simulink model "MCVT_Integration"  (R2020a)
settings: Solver=ode8  StartTime=0.0  StopTime=T_end  FixedStep=dt  RelTol=1e-3  EnableMultiTasking=on
values from neighbouring files (symbols this model uses):
  assumed_power_voltage = 1.25e2    (= 125)    [MCVT_Input_File.m]    (also in MCVT_Run_QuickCheck.m)
  battery_power_toggle = 1    [MCVT_Input_File.m]    (also in MCVT_Run_QuickCheck.m)
  battery_trickle_charge_param = 0.1    [MCVT_Input_File.m]    (also in MCVT_Run_QuickCheck.m)
  battery_trickle_leakage_param = 0.01    [MCVT_Input_File.m]    (also in MCVT_Run_QuickCheck.m)
  damage_case_id = 3    [MCVT_Input_File.m]    (also in MCVT_Run_QuickCheck.m = 1)
  dt = 1/fs    (= 0.001)    [MCVT_Run_QuickCheck.m]    (also in MCVT_Sim_Set.m)
  dt_2 = 1/fs_2    (= 0.001)    [MCVT_Run_QuickCheck.m]    (also in MCVT_Sim_Set.m)
  dt_3 = 1/fs_3    (= 0.001)    [MCVT_Run_QuickCheck.m]    (also in MCVT_Sim_Set.m)
  dt_5 = 1/fs_5    (= 0.005)    [MCVT_Run_QuickCheck.m]    (also in MCVT_Sim_Set.m)
  dt_8 = 1/fs_8    (= 0.05)    [MCVT_Run_QuickCheck.m]    (also in MCVT_Sim_Set.m)
  dt_9 = 1/fs_9    (= 0.001)    [MCVT_Run_QuickCheck.m]    (also in MCVT_Sim_Set.m)
  dust_met_duration = 3    [MCVT_Input_File.m]    (also in MCVT_Run_QuickCheck.m = 2)
  ECLSS_indoor_set_temperature = 298.15    [MCVT_Input_File.m]    (also in MCVT_Run_QuickCheck.m)
  energy_store_max_o = 150    [MCVT_Input_File.m]    (also in MCVT_Run_QuickCheck.m)
  energy_store_o = 120    [MCVT_Input_File.m]    (also in MCVT_Run_QuickCheck.m)
  fs = 1000    [MCVT_Run_QuickCheck.m]    (also in MCVT_Sim_Set.m)
  gain_charge_param = 1    [MCVT_Input_File.m]    (also in MCVT_Run_QuickCheck.m)
  ground_temperature = 373    [MCVT_Habitat_Design_Input_File.m]    (also in MCVT_Run_QuickCheck.m)
  I_max_charge = 800    [MCVT_Input_File.m]    (also in MCVT_Run_QuickCheck.m)
  I_max_discharge = 800    [MCVT_Input_File.m]    (also in MCVT_Run_QuickCheck.m)
  initial_pressure = 1e5    (= 100000)    [MCVT_Input_File.m]    (also in MCVT_Run_QuickCheck.m)
  initial_temperature = 295    [MCVT_Input_File.m]    (also in MCVT_Run_QuickCheck.m)
  interior_leak_rate = 0.00415    [MCVT_Input_File.m]    (also in MCVT_Run_QuickCheck.m)
  launch_dust_accum_start_time = 10    [MCVT_Input_File.m]    (also in MCVT_Run_QuickCheck.m = 2)
  launch_dust_accum_stop_time = 12    [MCVT_Input_File.m]    (also in MCVT_Run_QuickCheck.m = 3)
  meteorite_impact_time = 2    [MCVT_Input_File.m]    (also in MCVT_Run_QuickCheck.m = 1)
  nuclear_power_toggle = 1    [MCVT_Input_File.m]    (also in MCVT_Run_QuickCheck.m)
  print_interval = 30    [MCVT_Input_File.m]
  SOC_lower_thres = 0.5    [MCVT_Input_File.m]    (also in MCVT_Run_QuickCheck.m)
  SOC_upper_thres = 0.9    [MCVT_Input_File.m]    (also in MCVT_Run_QuickCheck.m)
  solar_power_toggle = 1    [MCVT_Input_File.m]    (also in MCVT_Run_QuickCheck.m)
  space_temperature = 4    [MCVT_Habitat_Design_Input_File.m]    (also in MCVT_Run_QuickCheck.m)
  Start_angle = 90    [MCVT_Input_File.m]    (also in MCVT_Run_QuickCheck.m)
undefined symbols (not defined in the file or in its folder): A_flow_restrictor, ACTIVITY_LOOKUP_TABLE, air, Aref, Bmap, Bmap2, Bmap3, comms, DiscIntNeverNeededParam, DmgSPL, dust_parameters, ECLSS_dust_damage_repair_time, ECLSS_heater_capacity, ECLSS_IPS_restrictor, ECLSS_IPS_setpointPressure, ECLSS_paint_damage_repair_time, ECLSS_radiatorLoop_discreteNumber, ECLSS_Vst, emptyActivity, expected_heat_add, FI, habitat, HIEM2SMM, HIEM2STM, HIEMDamageElements, impact_numbering, initial_SPLT_damage_index, input_air, input_Cond, input_CP, input_discretization, input_EV, input_Evap, input_radiatorLoop, K_ECLSS_dust_single, K_ECLSS_paint_single, K_Structure_damage_single, Kref, kW_to_W, meteoroidDustDep, mFRF, ndelays, nominalDustDep, noverlap, nuclear_toggle_gain, output_limits, panel_paint0, Ps0, reference_temperature, RepairTime_ECLSS_factor, Sensor_Location_SMM, SMM_T0, SMM_T1, SMM_T2, SMM_T3, SMM_T4, SMM_T4_0, SMM_T4_1, SMM_T4_2, solar_FDD_K ... (+73)
libraries: neural x350, simulink x39, xpcutilitieslib x7, xpcrtudplib x7, dspstat3 x1
(part 41 of 43)

== matlab function MCVT_Integration/Sys8 - Interior Environment/Habitat Interior Environment Model/Temperature Sensors/ Resistance Temperature Detector2/R vs T ==
  data: R input; T output
function T = fcn(R)

T = 1.76520388802152e-09 * R^4 - 2.98990866289637e-07 * R^3 + 0.000987249006463873 * R^2 ...
    + 2.36178487086887 * R - 245.924688605077;

end

== matlab function MCVT_Integration/Sys8 - Interior Environment/Habitat Interior Environment Model/Temperature Sensors/ Resistance Temperature Detector2/RTD with Self-heat Error/MATLAB Function ==
  data: I input; R input; U output
function U = fcn(I, R)

U = I * R;     % Operating current = 1mA
               % Voltage output U = I*R

== matlab function MCVT_Integration/Sys8 - Interior Environment/Habitat Interior Environment Model/Temperature Sensors/ Resistance Temperature Detector2/RTD without Self-heat Error/MATLAB Function ==
  data: I input; I output; R input; U output
function [I,U]= fcn(I,R)

U = I * R;     % Operating current = 1mA
               % Voltage output U = I*R

== matlab function MCVT_Integration/Sys8 - Interior Environment/Habitat Interior Environment Model/Temperature Sensors/ Resistance Temperature Detector2/Self-heat Calculation/  T vs R  ==
  data: R output; T input
function R = fcn(T)

if T >= 0
    R = 100*(1+3.9083*10^(-3)*T-5.775*10^(-7)*T^2);
else
    R = 100*(1+3.9083*10^(-3)*T-5.775*10^(-7)*T^2-4.183*10^(-12)*(T-100)*T^3);
end

== matlab function MCVT_Integration/Sys8 - Interior Environment/Habitat Interior Environment Model/Temperature Sensors/ Resistance Temperature Detector2/Switch ON//OFF Control Block/Switch OFF/MATLAB Function1 ==
  data: u input; y output; Time input
function y = fcn(u,Time)

if Time>=0
    u = NaN;
end

y = u;

== matlab function MCVT_Integration/Sys8 - Interior Environment/Habitat Interior Environment Model/Temperature Sensors/ Resistance Temperature Detector2/T vs R ==
  data: R output; T input
function R = fcn(T)

if T >= 0
    R = 100*(1+3.9083*10^(-3)*T-5.775*10^(-7)*T^2);
else
    R = 100*(1+3.9083*10^(-3)*T-5.775*10^(-7)*T^2-4.183*10^(-12)*(T-100)*T^3);
end

== matlab function MCVT_Integration/Sys8 - Interior Environment/Habitat Interior Environment Model/Temperature Sensors/ Switch ON//OFF Control Number ==
  data: Power input; Switch output
function Switch = Switch1(Power)

if Power == 0
    Switch = 2;
else
    Switch = 1;
end

end

== matlab function MCVT_Integration/Sys8 - Interior Environment/Habitat Interior Environment Model/Temperature Sensors/ Switch ON//OFF Control Number1 ==
  data: Power input; Switch output
function Switch = Switch2(Power)

if Power == 0
    Switch = 2;
else
    Switch = 1;
end

end

== matlab function MCVT_Integration/Sys8 - Interior Environment/Habitat Interior Environment Model/Temperature Sensors/ Switch ON//OFF Control Number2 ==
  data: Power input; Switch output
function Switch = Switch3(Power)

if Power == 0
    Switch = 2;
else
    Switch = 1;
end

end

== matlab function MCVT_Integration/Sys3 - Power System/Power Generation Subsystem1/Nuclear Power Generation/SP-100 Power Generation/	Power Generation/Sensor Models/Cold Junction Temperature Sensing Model4/ Resistance Temperature Detector/R vs T ==
  data: R input; T output
function T = fcn(R)

T = 1.76520388802152e-09 * R^4 - 2.98990866289637e-07 * R^3 + 0.000987249006463873 * R^2 ...
    + 2.36178487086887 * R - 245.924688605077;

end

== matlab function MCVT_Integration/Sys2 - Structural System/Sys2_Structural_System/Sim FDD Damage Detection/MATLAB Function ==
  data: u input; xtrue output
function xtrue = TobinaryHS_damage(u)
    if u > 0.5
        xtrue = 1;
    else
        xtrue = 0;
    end
end

== matlab function MCVT_Integration/Sys3 - Power System/Power Generation Subsystem1/Nuclear Power Generation/SP-100 Power Generation/	Power Generation/Sensor Models/Cold Junction Temperature Sensing Model4/ Resistance Temperature Detector/RTD with Self-heat Error/MATLAB Function ==
  data: I input; R input; U output
function U = fcn(I, R)

U = I * R;     % Operating current = 1mA
               % Voltage output U = I*R

== matlab function MCVT_Integration/Sys8 - Interior Environment/Habitat Interior Environment Model/Temperature Sensors/Sensor Input to CDCM ==
  data: ECLSS input; Air_Temp_Sensed output; Zenith input; LeftBottom input
function Air_Temp_Sensed = Sensor_Placement(ECLSS,Zenith,LeftBottom)

a = ECLSS(1,1);
b = Zenith(1,1);
c = LeftBottom(1,1);

Air_Temp_Sensed = ones(3,1);

Air_Temp_Sensed(1,1) = a;
Air_Temp_Sensed(2,1) = b;
Air_Temp_Sensed(3,1) = c;

end

== matlab function MCVT_Integration/Sys8 - Interior Environment/Habitat Interior Environment Model/Temperature Sensors/Sensor Placement ==
  data: Air_Temp input; ECLSS output; Zenith output; LeftBottom output
function [ECLSS,Zenith,LeftBottom] = Sensor_Placement(Air_Temp)

Locations = [65 10 19];

Temp_to_Sensor = Air_Temp(Locations,1);

ECLSS = Temp_to_Sensor(1,1);
Zenith = Temp_to_Sensor(2,1);
LeftBottom = Temp_to_Sensor(3,1);

end

== matlab function MCVT_Integration/Sys3 - Power System/Power Generation Subsystem1/Nuclear Power Generation/SP-100 Power Generation/	Power Generation/Sensor Models/Cold Junction Temperature Sensing Model4/ Resistance Temperature Detector/RTD without Self-heat Error/MATLAB Function ==
  data: I input; I output; R input; U output
function [I,U]= fcn(I,R)

U = I * R;     % Operating current = 1mA
               % Voltage output U = I*R

== matlab function MCVT_Integration/Sys3 - Power System/Power Generation Subsystem1/Nuclear Power Generation/SP-100 Power Generation/	Power Generation/Sensor Models/Cold Junction Temperature Sensing Model4/ Resistance Temperature Detector/Self-heat Calculation/  T vs R  ==
  data: R output; T input
function R = fcn(T)

if T >= 0
    R = 100*(1+3.9083*10^(-3)*T-5.775*10^(-7)*T^2);
else
    R = 100*(1+3.9083*10^(-3)*T-5.775*10^(-7)*T^2-4.183*10^(-12)*(T-100)*T^3);
end

== matlab function MCVT_Integration/Sys3 - Power System/Power Generation Subsystem1/Nuclear Power Generation/SP-100 Power Generation/	Power Generation/Sensor Models/Cold Junction Temperature Sensing Model4/ Resistance Temperature Detector/Switch ON//OFF Control Block/Switch OFF/MATLAB Function1 ==
  data: u input; y output; Time input
function y = fcn(u,Time)

if Time>=0
    u = NaN;
end

y = u;

== matlab function MCVT_Integration/Sys3 - Power System/Power Generation Subsystem1/Nuclear Power Generation/SP-100 Power Generation/	Power Generation/Sensor Models/Cold Junction Temperature Sensing Model4/ Resistance Temperature Detector/T(Celcuis) vs R ==
  data: R output; T input
function R = fcn(T)

if T >= 0
    R = 100*(1+3.9083*10^(-3)*T-5.775*10^(-7)*T^2);
else
    R = 100*(1+3.9083*10^(-3)*T-5.775*10^(-7)*T^2-4.183*10^(-12)*(T-100)*T^3);
end

== matlab function MCVT_Integration/Sys3 - Power System/Power Generation Subsystem1/Nuclear Power Generation/SP-100 Power Generation/	Power Generation/Sensor Models/block/MATLAB Function2 ==
  data: y_Tf output; y_process_scale_lower input; y_Tb1 output; y_Tb2 output; y_Tc2 output
function [y_Tf, y_Tb1, y_Tb2, y_Tc2] = fcn(y_process_scale_lower)
    y_Tf = y_process_scale_lower(:,1);
    y_Tb1 = y_process_scale_lower(:,2);
    y_Tb2 = y_process_scale_lower(:,3);
    y_Tc2 = y_process_scale_lower(:,4);
end

== matlab function MCVT_Integration/Sys3 - Power System/Power Generation Subsystem1/Solar Power Generation/MATLAB Function1 ==
  data: u input; y output
function y = ToTrueHealth(u)
    if 0 <= u && u < 0.05
        y = 1; % healthy
    elseif 0.05 <= u && u < 0.3
        y = 2; % minor
    elseif 0.3 <= u && u < 0.7
        y = 3; % medium
    else %0.7 <= u && u <= 1
        y = 4; % major
    end

== matlab function MCVT_Integration/Sys3 - Power System/Power Generation Subsystem1/Nuclear Power Generation/SP-100 Power Generation/FDD Iterative Online Residual Analysis1/ Digital Twin of SP100/Residual Generation/Compare to Nominal/CUSUM5/cusum ==
  data: intervention input; cusum_stat1 output; time input; time_step input; cusum_stat1 input; intervention_previous output; intervention_previous input
function [cusum_stat1,intervention_previous]  = fcn(intervention, time, time_step, cusum_stat1,intervention_previous)

[cusum_stat1,intervention_previous]  = cusum(intervention, time, time_step, cusum_stat1,intervention_previous);

end

== matlab function MCVT_Integration/Sys3 - Power System/Power Generation Subsystem1/Nuclear Power Generation/SP-100 Power Generation/FDD Iterative Online Residual Analysis1/ Digital Twin of SP100/Residual Generation/FDD_toggle ==
  data: FDD_signal input; FDD_signal_sent output; nuclear_power_toggle input; battery_power_toggle input
function FDD_signal_sent = FDD_toggle(FDD_signal, nuclear_power_toggle,...
    battery_power_toggle)

FDD_signal_sent = FDD_toggle(FDD_signal, nuclear_power_toggle,...
    battery_power_toggle);

end

== matlab function MCVT_Integration/Sys3 - Power System/Power Generation Subsystem1/Power Distribution and Storage System/Subsystem/power_gen_allocation ==
  data: G_solar_temporary input; G_solar_power output; P_out_solar input; V input; I_tot input; energy_stored input; max_storage input; time_step input; G_nuclear input; I_nuclear input; SOC_lower_thresh input; energy_stored output; time_to_discharge output; SOC_upper_thresh input; SOC output; G_nuclear_power output; battery_use_status output; leakage_parameter input; trickle_charge_parameter input; charge_parameter input; I_max_charge input; I_max_discharge input; total_power_avail output; load_shed_status output; I_battery output; sub2_request input; sub5_request input; sub8_request input; battery_power_toggle input
function [G_solar_power,energy_stored,time_to_discharge,SOC,...
    G_nuclear_power,battery_use_status,total_power_avail,...
    load_shed_status,I_battery]=power_gen_allocation(G_solar_temporary,...
    P_out_solar,V,I_tot,energy_stored,max_storage,time_step, G_nuclear,...
    I_nuclear,SOC_lower_thresh,SOC_upper_thresh, leakage_parameter,...
    trickle_charge_parameter,charge_parameter,I_max_charge,...
    I_max_discharge,sub2_request,sub5_request,sub8_request,...
    battery_power_toggle)


[G_solar_power,energy_stored,time_to_discharge,SOC,...
    G_nuclear_power,battery_use_status,total_power_avail,...
    load_shed_status,I_battery]=power_gen_allocation(G_solar_temporary,...
    P_out_solar,V,I_tot,energy_stored,max_storage,time_step, G_nuclear,...
    I_nuclear,SOC_lower_thresh,SOC_upper_thresh, leakage_parameter,...
    trickle_charge_parameter,charge_parameter,I_max_charge,...
    I_max_discharge,sub2_request,sub5_request,sub8_request,...
    battery_power_toggle);


end

== matlab function MCVT_Integration/Sys3 - Power System/Power Generation Subsystem1/Power Distribution and Storage System/Subsystem/MATLAB Function1 ==
  data: I_nuc input; G_nuc output; I_tot input
function G_nuc  = fcn(I_nuc,I_tot)
if I_nuc ~= 0
    G_nuc = min(1, I_tot/I_nuc);
else
    G_nuc = 0;
end
end

== matlab function MCVT_Integration/Sys3 - Power System/Power Generation Subsystem1/Power Distribution and Storage System/Subsystem2/Dummy Electrical Power Consuming Equipment3/MATLAB Function ==
  data: p_avail input; p_desired output
function p_desired  = fcn(p_avail)

  % A system that has two modes and enters mode 2 if p_avail < 20
  if (p_avail < 20)
        p_desired = p_avail;
  else
        p_desired = 50;
  end

end

== matlab function MCVT_Integration/Sys2 - Structural System/Sys2_Structural_System/Structural Thermal Model 2.2/STM Temperature Sensors/ Resistance Temperature Detector/Power Consumption ==
  data: Supply input; kWUsage1 output; Switch input
function kWUsage1 = Power_Consumption(Supply,Switch)
if Switch >= 1
    Voltage = 5.5; %Volts
    Current = 0.000001; %10 microAmperes
    kWUsage1 = ones(1,1);
    
    kWUsage1 = (Voltage * Current)/1000;
    
    if kWUsage1 >= Supply
        kWUsage1 = Supply;
    end
else
    kWUsage1 = 0;
end
end

== matlab function MCVT_Integration/Sys3 - Power System/Power Generation Subsystem1/printRepair ==
  data: x input
function  printRepair(x)


if x(1)>0
    disp('        Solar Panel is Cleaned');
end   


end

== matlab function MCVT_Integration/Sys3 - Power System/Power Generation Subsystem1/printRepair1 ==
  data: x input
function printRepair(x)


if x(1)>0
    disp('        Nuclear Radiator is Cleaned');
end   


end

== matlab function MCVT_Integration/Sys2 - Structural System/Sys2_Structural_System/Fault Detection and Diagnosis (FDD) 2.3/Accelerometer/Accelerometer/ Switch ON//OFF Control Number ==
  data: p input; y output
function y = fcn(p)

if p >= 1.2*10^(-4)*3
    u = 1;
else
    u = 2;
end

y = u;

== matlab function MCVT_Integration/Sys8 - Interior Environment/power1 ==
  data: Y output; x input
function Y = poweredit(x)

Y=max(5.5e-9*3, x);

end

== matlab function MCVT_Integration/Sys2 - Structural System/Sys2_Structural_System/Structural Mechanical 2.1/MATLAB Function ==
  data: u input; y output
function y = fcn(u)

y = norm(u);

end

== matlab function MCVT_Integration/Sys2 - Structural System/power2 ==
  data: Y output; x input
function Y = poweredit(x)

Y=max(0.0036, x);

end

== matlab function MCVT_Integration/Sys9 - Exterior Environment/Exterior Environment/Reads cartesian coordinates ==
  data: pos_out output
function pos_out = Coordinate_reader()
    
CARCOOM=coder.load ('SPLxyz.mat'); %Loads the user provided cartesian coordinates into the program%
pos_out=CARCOOM.STMregxyz;

end

== matlab function MCVT_Integration/Sys5 - ECLSS/Human//System 5/Human Heat Model V2 ==
  data: dt input; activity_id input; heat_profile_final output; Location_id input; r output; theta output
function [heat_profile_final, r, theta]  =...
    eclss_human_model(dt,activity_id,Location_id)


%Activity 1: SleepHeat = 317 kJ/hr
%Activity 2: NominalHeat = 500 kJ/hr
%Activity 3: Exercise1 = 1206 kJ/hr
%Activity 4: Exercise2 = 2974 kJ/hr
%Activity 5: Exercise3 = 2005 kJ/hr
%Activity 6: Exercise4 = 1078 kJ/hr
%Activity 7: Exercise5 = 865 kJ/hr
%Activity 8: Exercise6 = 751 kJ/hr
%Activity 9: Other = 0 kJ/hr


activity_heat = [317 500 1206 2974 2005 1078 865 751 0]; %kJ/hr
heat_per_hr = activity_heat(floor(activity_id)); %kJ/hr
heat_profile = heat_per_hr/(60*60)*dt*1000; %J/dt
heat_profile_final = heat_profile * Location_id(1);
r = Location_id(2); % feet
theta = Location_id(3); % degrees

== matlab function MCVT_Integration/Sys 6 - Health Management and Agents/Decision Maker/MATLAB Function ==
  data: suggested_activity_id input; pgm output; lookup_table input; time input
function pgm = acvt_lookup_function(suggested_activity_id, lookup_table, time)
%         #=================================================#
%         #    Code developed for the RETH-Institute        #
%         #    By: Murali Krishnan Rajasekharan Pillai      #
%         #    PhD Student @ Purdue University              #
%         #    Funded by: NASA                              #
%         #    Last Modified: July 29, 2021                 #
%         #=================================================#
% 
%         Purpose: Function that looks-up activity definitions from a look-up table
%         specified in the `InitAgentModel.m`

    valid_activity_ids = [1, 2, 3, 4, 5, -1];
    valid_active_acvt_ids = [1, 2, 3, 4, 5];
    assert(ismember(suggested_activity_id, valid_activity_ids), "Invalid Activity ID");
    if ismember(suggested_activity_id, valid_active_acvt_ids)
        pgm = squeeze(lookup_table(suggested_activity_id, :, :));
    else
        pgm = squeeze(lookup_table(6, :, :));
    end
    
    if time == 35 && suggested_activity_id == -9999
        pgm = squeeze(lookup_table(4, :, :));
    end
    if time == 30 && suggested_activity_id == -9999
        pgm = squeeze(lookup_table(5, :, :));
    end
end

== matlab function MCVT_Integration/Sys2 - Structural System/Sys2_Structural_System/Fault Detection and Diagnosis (FDD) with Binary Classifer/Post-processing Block ==
  data: u input; y output; t input; repair_begin input; impact_begin input
function y = fcn(u,t,repair_begin,impact_begin)

persistent count repair_begin_time

if isempty(count) && isempty(repair_begin_time)
    count = 0;
    repair_begin_time = 10000;
end

if ~isnan(repair_begin) && count == 0
    repair_begin_time = repair_begin;
    count = 1;
end

if t >= 0 && t < 7 + 0.002
    u(:,:) = nan;
else
    if t >= impact_begin + 0.002 && t < impact_begin + 7 + 0.002
        u(:,:) = nan;
    else
        if t >= ceil(repair_begin_time) + 0.002 && t < ceil(repair_begin_time) + 7 + 0.002
            % for impact location [-2.4807    1.0619    1.0622]
            % repair happen 39.4140s, reapir complete 40.4130
            % for other impact locations
            % repair happen 33.4120s, reapir complete 34.4110
            u(1:8,:) = nan;
        end
    end
end

% For segment 5
if t >= ceil(repair_begin_time) + 0.002 && t < ceil(repair_begin_time) + 13 + 0.002
    u(9:10,:) = nan;
end
            
y = u;

== matlab function MCVT_Integration/Sys2 - Structural System/Sys2_Structural_System/Fault Detection and Diagnosis (FDD) with Binary Classifer/Find repair start time ==
  data: u input; y output; t input
function y = fcn(u,t)

persistent count

if isempty(count)
    count = 0;
end

if u == 1 && count == 0
    y = t;
    count = 1;
else
    y = nan;
    
end

== matlab function MCVT_Integration/Sys5 - ECLSS/Active Thermal Control System (ATCS)/Faulty Panels/MATLAB Function3 ==
  data: impact_numbering input; N input; impact input; L_dust output size=[1,50]
function L_dust  = Damage_factory_Dust(impact_numbering, N, impact)
%% Documentation
% Purpose: Assign which panels to be damaged when there is a meteorite
% impact
% Date Created: 20 June 2020
% Date Last Modified: 27 July 2021
% Modeler Name: Jaewon Park (Purdue)
% Funding Acknowledgement: Funded by the NASA RETHi Project (80NSSC19K1076)

% Version Number: MCVT v1.4

% Subsystem Connections: receives input from subsystem 8.

% INPUTS %
%   - impact_numbering: impact scenario lower and upper bounds, type: integer, [1x2]
%   - impact: impact severity, type: integer
%   - N: total number of panel subsections, type: integer
    
% OUTPUTS %
%   - L_dust: list of subsection indices which should be damaged, [1x50], type: integer 

% Function Dependencies: No dependency

% No Data Depenencies
%% assign damaged panels by meteorite impact 
min_impact = impact_numbering(1);                                                                     % minimum impact severity         
max_impact = impact_numbering(2);                                                                     % maximum impact severity
n_panels_impact = 20;                                                               % maximum number of panels that can be impacted (To do) update needed as we move on from 60s simulation limit
mapping_ratio_impact = (impact - min_impact)/max_impact;                            % mapping 
n_to_be_affected_impact = round(n_panels_impact * mapping_ratio_impact);            % number of panels to be affected 
%disp(n_to_be_affected_impact)
temp = sort([randperm(N,n_to_be_affected_impact), zeros(1,N-n_to_be_affected_impact)],'descend');   
L_dust = zeros(1,50);
L_dust = L_dust + temp;                                                             % assign damaged panels
end

== matlab function MCVT_Integration/Sys2 - Structural System/Sys2_Structural_System/Structural Mechanical 2.1/Force Calculation ==
  data: Ext_F output; In_Acc output; fT input; fT_e input; eT input; TIn input; Dom input; PIn input; MQ_Acc input; WN_Acc input; Imp_F input; T_Node input; SMM_T0 parameter; SMM_T1 parameter; fTO output; fT_eO output; eTO output; SMM_T2 parameter; SMM_T4_0 parameter; SMM_T4_1 parameter; SMM_T4_2 parameter
function [Ext_F,In_Acc,fTO,fT_eO,eTO]=Force_Calculation_Sim(fT,fT_e,eT,TIn,Dom,PIn,MQ_Acc,WN_Acc,Imp_F,T_Node,SMM_T0,SMM_T1,SMM_T2,SMM_T4_0,SMM_T4_1,SMM_T4_2)
            

%% This block determine Equivalent force vector from 
%   1. Internal Pressure
%   2. Temperature
%   3. Ground acceleration and White noise
%   4. Impact force

% Inputs from outher models:
%       PIn: Pressure input (Interior env)
%       TIn: Temperature input from STM (profile for Structure)
%       In_Acc: Acceleration Input
%       Imp_F: Impact force input

% Inputs from Main\Workspace
%       SMM_T0,SMM_T1,SMM_T2,SMM_T4_0,SMM_T4_1,SMM_T4_2: Read SysFiles\Subs2\SMM_Readme.txt

% State dependent var for SMM computation:
%       fT: Force vector due to temperature at previous timestep/iteration
%       fT_e: Force matrix (per element) due to temperature at previous timestep/iteration
%       eT: Element number for thermal stress computation at previous timestep/iteration
%       Dom: Domain number

% Outputs:
%       Ext_F: Force vector output to Solver block
%       Ext_A: Acceleration vector output to Solver block
%       fTO: Force vector due to temperature at this timestep/iteration
%       fT_eO: Force matrix (per element) due to temperature at this timestep/iteration
%       eTO: Element no for thermal stress computation at this timestep/iteration

% System D.O.F.
    ndof=3;

  
%% Equivalent nodal force from Internal pressure calculation (High efficiency)

    % Get pressure by averaging internal pressure PIn from Interior environment model
        P=zeros(SMM_T1.nel,1)+mean(PIn);   
            
    % Calculate Force vector due to pressure
        [fS]=Pressure_to_Equivalent_Nodal_Force(SMM_T1.nnp,SMM_T4_1.LM_M(:,:,Dom),SMM_T0.Type,SMM_T0.S_Type,SMM_T2.P_surf,P); % Convert pressure to Nodal force 
          
            
%% Equivalent nodal force from Temperature calculation (Low efficiency) 

    % Summary
        % Thermal stress computation is computationally extensive. 
        % Hence thermal stress for one element "eT" per iteration has been carried out

    % ---------------Construct temperature using mapping vector (One to One)-------------
        % Safe: Can work with any domain
        % STM map temperature for Domain 1. Nodes for Domain 1
            NodesFP=SMM_T4_0.Nodes_M(:,:,1); 
        % Nodes for Domain Dom
            Nodes=SMM_T4_0.Nodes_M(:,:,Dom);
        % Initialize Temperature for Domain Dom    
            T=zeros(SMM_T1.nnp,1)+nanmean(TIn); % Initialize Temperature vector with mean temperature
            
            for n=1:SMM_T1.nnp % Go through all nodes
                for ii=1:SMM_T1.nnp
                    % Distance between Node n of Domain Dom and Node i of Domain NodesFP
                    Diff=norm(Nodes(n,2:4)-NodesFP(ii,2:4));  
                    if abs(Diff)<10^-5  % If difference <Tol
                        if isnan(TIn)==0 % Safety check for input loadings if they become NaN
                            T(n)=TIn(ii);    % Set the Temperature value
                        end
                        break
                    end
                end
            end
                
    % ----------------------------Convert Nodal Temperature to Gauss point-----------------------
        [Tm]=Gauss_Point_Temperature_Matrix_Generator(T,SMM_T0.Type,SMM_T1.Shape,SMM_T4_1.IEN_Colm_M(:,Dom),SMM_T1.nel);   
            
    % -------------------Convert Gauss point temperature to Equivalent nodal force-----------------
        % Subtract the elemental Force vector of element eT from assembled force vector fT
            fT(SMM_T4_1.LM_M(1:SMM_T0.Type(2,eT)*ndof,eT,Dom),1)=fT(SMM_T4_1.LM_M(1:SMM_T0.Type(2,eT)*ndof,eT,Dom),1)-fT_e(1:SMM_T0.Type(2,eT)*ndof,eT);   % Eleminate force due to Temperature for element eT from total force vector fT                    
        % Initialize the elemental force vector for eT     
            fT_e(:,eT)=fT_e(:,eT)*0;     % Initialize FV. Assign 0 at local force vector fT_e for element eT
               
        % Get fT_e, Force vector due to temperature for element eT
            for gp=1:SMM_T0.Type(ndof,eT)
                % fT_e=fT_e+IS_F*delT
                fT_e(1:SMM_T0.Type(2,eT)*ndof,eT)=fT_e(1:SMM_T0.Type(2,eT)*ndof,eT)+SMM_T2.IS_F(1:SMM_T0.Type(2,eT)*ndof,gp,eT)*(Tm(gp,eT)-SMM_T0.Tinit);               
            end
        % Add fT_e to assembled force vector fT
            fT(SMM_T4_1.LM_M(1:SMM_T0.Type(2,eT)*ndof,eT,Dom),1)=fT(SMM_T4_1.LM_M(1:SMM_T0.Type(2,eT)*ndof,eT,Dom),1)+fT_e(1:SMM_T0.Type(2,eT)*ndof,eT);

        % If eT is higher than SMM_T1.nel, reset eT
            eT=eT+1;
            if eT>SMM_T1.nel
               eT=1;
            end

        % Setting output different name then input
            fTO=fT;
            fT_eO=fT_e;
            eTO=eT;

%% Total force vector calculation

    % fT: Thermal
    % SMM_T4_2.fB_M: Gravity
    % fS: Internal pressure
    % L is the D.O.F. left after removing Fixed end condition

    Ext_F=(fT+SMM_T4_2.fB_M(:,Dom)+fS);

%% Equivalent nodal force from Impact force calculation      
    % Convert Impact force perpendicular to node

    % Set the impact force
    if Imp_F>0
        % Normal unit vector of the surface
            Rad=norm([SMM_T4_0.Nodes_M(T_Node,2,Dom) SMM_T4_0.Nodes_M(T_Node,ndof,Dom) SMM_T4_0.Nodes_M(T_Node,4,Dom)]);
        % Set the impact force
            Imp_F=-Imp_F/Rad;
            Ext_F(T_Node*ndof-2,1)=Ext_F(T_Node*ndof-2,1)+Imp_F*SMM_T4_0.Nodes_M(T_Node,2,Dom);
            Ext_F(T_Node*ndof-1,1)=Ext_F(T_Node*ndof-1,1)+Imp_F*SMM_T4_0.Nodes_M(T_Node,ndof,Dom);
            Ext_F(T_Node*ndof,1)=Ext_F(T_Node*ndof,1)+Imp_F*SMM_T4_0.Nodes_M(T_Node,4,Dom);            
    end
            

%% Acceleration computation     

    % Initialize acceleration
        In_Acc=zeros(SMM_T1.neq,1);

    % Set acceleration vector along x direction    
        In_Acc(1:ndof:end-2,1)=ones(SMM_T1.neq/ndof,1)*(MQ_Acc(1)+WN_Acc(1));
    % Set acceleration vector along y direction
        In_Acc(2:ndof:end-1,1)=ones(SMM_T1.neq/ndof,1)*(MQ_Acc(2)+WN_Acc(1));
    % Set acceleration vector along z direction
        In_Acc(3:ndof:end-0,1)=ones(SMM_T1.neq/ndof,1)*(MQ_Acc(3)+WN_Acc(1));            
            
 
end

%% Pressure calculation
function[fS]=Pressure_to_Equivalent_Nodal_Force(nnp,LM,Type,S_Type,P_surf,Ps)

% S_Type contains surface information
    SS=size(S_Type);

% Initialize force vector due to pressure on surface fS        
    fS=zeros(nnp*3,1);

% For all the surfaces
    for ii=1:SS(1)

        SN=S_Type(ii,1);                           % Extract Surface number

        e=S_Type(ii,2);                            % Extract element no

        Pes=Ps(ii,SN);                             % Extract Element Pressure

        fe=P_surf(1:Type(2,e)*3,ii,SN)*Pes;        % Determine Equivalent Nodal Force vector

        fS(LM(1:Type(2,e)*3,e))=fS(LM(1:Type(2,e)*3,e))+fe;     % Put into Total/assembled Force Vector

    end
    

end

%% Gauss point temperature calculation 
function[Tm]=Gauss_Point_Temperature_Matrix_Generator(T,Type,Shape,IEN_Colm,nel)

% Temperature in Expanded format
    Te=T(IEN_Colm);
% Initialize temperature matrix 
    % Rows: Gauss points
    % Column: Element number
    Tm=zeros(max(Type(3,:)),nel);

% Populate Tm for each element    
    count=1;
    for e=1:nel % Element number e

        % Get Temperature at Gauss points for specific element e
            Tm(1:Type(3,e),e)=Shape(1:Type(3,e),1:Type(2,e),Type(1,e))*Te(count:count+Type(2,e)-1,1); 

        % Keep track of Expanded temperature vector
            count=count+Type(2,e);
    end


end

== matlab function MCVT_Integration/Sys3 - Power System/Power Generation Subsystem1/accumulation adjust ==
  data: x input; y output
function y = dust_accumu(x)

y=max(x*500,0);


end

== matlab function MCVT_Integration/Sys3 - Power System/Power Generation Subsystem1/Power Distribution and Storage System/Power Delivered to each EPCE/power_distribution ==
  data: total_power_avail input; power_demands output; power_demands input; status_data output
function [power_demands, status_data]  = power_distribution(...
     total_power_avail,power_demands)

[power_demands, status_data]  = power_distribution(...
     total_power_avail,power_demands);
 
end

== matlab function MCVT_Integration/Sys5 - ECLSS/Active Thermal Control System (ATCS)/Faulty Panels/MATLAB Function4 ==
  data: impact_numbering input; N input; impact input; L_paint output size=[1,50]
function L_paint  = Damage_factory_paint(impact_numbering, N, impact)
%% Documentation
% Purpose: Assign which panels to be damaged when there is a meteorite
% Date Created: 20 June 2020
% Date Last Modified: 27 July 2021
% Modeler Name: Jaewon Park, CJ Pan (Purdue)
% Funding Acknowledgement: Funded by the NASA RETHi Project (80NSSC19K1076)

% Version Number: MCVT v1.4

% Subsystem Connections: receives input from subsystem 8.

% INPUTS %
%   - impact_numbering: impact scenario lower and upper bounds, type: integer, [1x2]
%   - impact: impact severity, type: integer
%   - N: total number of panel subsections, type: integer
    
% OUTPUTS %
%   - L_paint: list of subsection indices which should be damaged, [1x50], type: integer

% Function Dependencies: No dependency

% No Data Depenencies
%% Assign paint damage
min_impact = impact_numbering(1);             % minimum impact severity 
max_impact = impact_numbering(2);             % maximum impact severity 
n_panels_impact = 20;       % maximum number of panels that can be impacted (To do) update needed as we move on from 60s simulation limit
mapping_ratio_impact = (impact - min_impact)/max_impact;    % mapping
n_to_be_affected_impact = round(n_panels_impact * mapping_ratio_impact);    % number of panels to be affected 
%disp(n_to_be_affected_impact)
temp = sort([randperm(N,n_to_be_affected_impact), zeros(1,N-n_to_be_affected_impact)],'descend');
L_paint = zeros(1,50);
L_paint = L_paint + temp;   % assign damaged panels
end

== matlab function MCVT_Integration/Sys5 - ECLSS/Active Thermal Control System (ATCS)/Faulty Panels/Thermal paint damage ==
  data: L_paint input; panel_paint0 input; panel_paint output
function panel_paint = paint_damage(L_paint, panel_paint0)
%% Documentation
% Purpose: Update panel parameters based on paint damage
% Date Created: 20 June 2020
% Date Last Modified: 27 July 2021
% Modeler Name: Jaewon Park, CJ Pan (Purdue)
% Funding Acknowledgement: Funded by the NASA RETHi Project (80NSSC19K1076)

% Version Number: MCVT v1.4

% Subsystem Connections: No connection.

% INPUTS %
%   - L_paint: list of damaged panel by paint, [1x50], type: integer
%   - panel_paint0: healthy panel property, [2x50], type: integer
    
% OUTPUTS %
%   - panel_paint: panel parameters with paint damage, [2x50], type: integer

% Function Dependencies: No dependency

% No Data Depenencies
%% update panel subsection material info based on damage
if L_paint == 0     % 0 means no panel damage at the given point 
    panel_paint = panel_paint0;     % rest all the panels to healthy state 
else
    L_paint = L_paint(L_paint ~= 0); % remove zeros
    % L_paint: Unique panels with damaged thermal paint
    M2=length(L_paint);
    
    % Damaged radiator thermal paint
    epsilon_dp=0.7;        % Emissivity of the damaged thermal paint
    alpha_dp=0.4;          % Absorptivity of the damaged thermal paint
    
    epsilon_p = panel_paint0(1,:);    % initialize with healthy emissivity
    alpha_p = panel_paint0(2,:);      % initialize with healthy absorptivity
    N=length(epsilon_p);            
    
    %% Some radiator panels have damaged thermal paint:
    for k=1:M2
        epsilon_p(L_paint(k))=epsilon_dp;   % change emissivity for dirty panels 
        alpha_p(L_paint(k))=alpha_dp;       % change absorptivity for dirty panels
    end
    
    panel_paint=zeros(2,N);                 
    panel_paint(1,:)=epsilon_p(:);      % make a matrix
    panel_paint(2,:)=alpha_p(:);
  

end

end

== matlab function MCVT_Integration/Sys5 - ECLSS/Active Thermal Control System (ATCS)/Faulty Panels/Sim-FDD-ECLSS-Dust/MATLAB Function2 ==
  data: x input; y output
function y = Tobinaryhealthstate_vec_dust(x)
    y = zeros(50,1);
    y(x(x ~= 0)) =1;

== matlab function MCVT_Integration/Sys5 - ECLSS/Active Thermal Control System (ATCS)/Faulty Panels/Sim-FDD-ECLSS-Paint/MATLAB Function6 ==
  data: x input; y output
function y = Tobinaryhealthstate_vec_paint(x)
    y = zeros(50,1);
    y(x(x ~= 0)) =1; 
end

== matlab function MCVT_Integration/Sys5 - ECLSS/Active Thermal Control System (ATCS)/on and off dynamic enforcer ==
  data: Pe input; Pc input; Vop output
function Vop = on_off_dynamic(Pe, Pc)
%% Documentation
% Purpose: smooth transition for extreme cases/heat pump failure
% Date Created: 20 June 2020
% Date Last Modified: 27 July 2021
% Modeler Name: Jaewon Park, CJ Pan (Purdue)
% Funding Acknowledgement: Funded by the NASA RETHi Project (80NSSC19K1076)

% Version Number: MCVT v1.4

% Subsystem Connections: No connection.

%%% All inputs and outputs to this function are 1 dimensional %%%
% INPUTS %
%   - Pe: evaporator pressure, unit: bar, type: float
%   - Pc: condensor pressure, unit: bar, type: float
    
% OUTPUTS %
%   - Vop: heat coefficient manager, type: float

% Function Dependencies: No dependency

% No Data Depenencies


if Pc<=Pe                                   % if evaporator pressure is higher than condensor pressure
    Vop=0;                                  % force the condenser heat transfer to be zero
elseif Pc>Pe && Pc<1.1*Pe                   % if condensor pressure is higher than evaporator but not that high
    Vop=20000*exp(1/(1-Pc/Pe));             % start choking the condensor performance,...
                                            % 20000 is a random big number that was chosen ...
                                            % to mimic the on/off dynamic of refrigeration cycle.
                                            % This number may need update
                                            % if design dimension changes
                                            % (There is not really analytic way to solve for this value.)
                                          
else
    Vop=1;                                  % otherwise, just let it be
end



end

== matlab function MCVT_Integration/Signal Selection2/Subsystem/MATLAB Function ==
  data: Repair_Comms_ECLSS input; Repair_ECLSS_Dust output; Repair_ECLSS_Paint output
function [Repair_ECLSS_Dust, Repair_ECLSS_Paint] = failure_mode_selection(Repair_Comms_ECLSS)
   persistent repair_dust;
   persistent repair_paint;
   nones = [0, 0, 0, 0]';
   if isempty(repair_dust) || isempty(repair_paint)
       repair_dust = nones;
       repair_paint = nones;
   end
   
   if ~isempty(Repair_Comms_ECLSS)
       failure_mode = Repair_Comms_ECLSS(1);
       if failure_mode == 1
           repair_dust = Repair_Comms_ECLSS;
           repair_paint = nones;
       else
           repair_dust = nones;
           repair_paint = Repair_Comms_ECLSS;
       end
   end
   Repair_ECLSS_Dust = repair_dust;
   Repair_ECLSS_Paint = repair_paint;

end

== stateflow chart MCVT_Integration/Sys 6 - Health Management and Agents/ Agent System/Agent Module/Action Scheduler/Stateflow Module (re-factored) ==
  note: This module outputs the appropriate parameters required to drive the Agent Plant model.
  state CheckAction
    en, du:
    currAcvtDone=0;
    currActDone=0;
  state DoAction
    en:
    if (idx <= numValidActions)
    [actID, reqSteps, actionParams, actSampleTime] = initializeNewAction(idx);
    else
    actID = EMPTY;
    actionParams = EMPTY*ones(size(actionData(1,4:end)' ,1), 1);
    currAcvtDone=1;
    end
    du:
    doSteps = doSteps + 1;
    currActDone=0;
    if doSteps == reqSteps - 1
    currActDone=1;
    end
    ex:
    numActionsDone=numActionsDone+1;
  (default) -> CheckAction
  DoAction -> DoAction [order 2] : [doSteps == reqSteps-1]
      | /{idx=idx+1;
      | doSteps=0;
      | currActDone=0;}
  CheckAction -> DoAction : [actionData(1,1) > 0]
      | /{
      | idx=1;
      | doSteps=0;
      | numActionsDone=0;
      | }
  DoAction -> CheckAction [order 1] : [(abs(actID-EMPTY) < 1e-5)]
      | {idx=1;
      | numActionsDone=0;
      | doSteps=0;
      | currActDone=0;
      | actSampleTime=0;}
  data: indx function_input; ID function_output; steps function_output; params function_output; reqTime function_output; actID output =EMPTY; actionParams output size=[numMaxActionParams-paramStartIndex+1 1] =EMPTY * ones(numMaxActionParams-paramStartIndex+1,1); actionData input size=[numMaxActions numMaxActionParams]; numValidActions input; reqSteps local; doSteps local; numActionsDone local; EMPTY constant double =EMPTY; dt constant double =dt_6; currAcvtDone output; currActDone output; actSampleTime output =0; idx local
  function [ID, steps, params, reqTime] = initializeNewAction(indx):
    | %         #=================================================#
    | %         #    Code developed for the RETH-Institute        #
    | %         #    By: Murali Krishnan Rajasekharan Pillai      #
    | %         #    PhD Student @ Purdue University              #
    | %         #    Funded by: NASA                              #
    | %         #    Last Modified: July 29, 2021                 #
    | %         #=================================================#
    | %     
    | function [ID, steps, params, reqTime] = initializeNewAction(indx)
    |     % Decode information from inputs
    |     ID = actionData(indx,1);
    |     modeTime = actionData(indx,2);
    |     spreadTime = actionData(indx, 3);    
    |     % Sample time
    |     reqTime = sampleTriangular(modeTime, spreadTime);
    |     steps = cast(reqTime/dt, 'int32');
    |     params = actionData(indx,4:end)';
    | end
    | 
    | function time = sampleTriangular(modeT, spreadT)
    | %   Sample from a triangular distribution
    | %   Ref: Triangular Distribution @ Wikipedia
    | %   (https://en.wikipedia.org/wiki/Triangular_distribution)
    | %   Input Arguments:
    | %       modeT       :   float
    | %                       Mode of the time taken by the agent to perform the
    | %                       current task
    | %       spreadT     :   float
    | %                       Expected spread of time for the agent to perform
    | %                       the current task
    | 
    |     lowerT = max(0, modeT - spreadT);
    |     upperT = modeT + spreadT;
    |     f_c = (modeT - lowerT) / (upperT - lowerT);
    |     u = rand;
    |     
    |     if (u >= 0) && (u < f_c)
    |         time = lowerT + sqrt(u * (upperT - lowerT) * (modeT - lowerT));
    |     else
    |         time = upperT - sqrt( (1 - u) * (upperT - lowerT) * (upperT - modeT));
    |     end
    | end

== matlab function MCVT_Integration/Sys2 - Structural System/Sys2_Structural_System/Structural Thermal Model 2.2/STM Temperature Sensors/ Resistance Temperature Detector/RTD with Self-heat Error/MATLAB Function ==
  data: I input; R input; U output
function U = fcn(I, R)

U = I * R;     % Operating current = 1mA
               % Voltage output U = I*R

== matlab function MCVT_Integration/Sys2 - Structural System/Sys2_Structural_System/Structural Thermal Model 2.2/STM Temperature Sensors/ Resistance Temperature Detector/RTD without Self-heat Error/MATLAB Function ==
  data: I input; I output; R input; U output
function [I,U]= fcn(I,R)

U = I * R;     % Operating current = 1mA
               % Voltage output U = I*R

== matlab function MCVT_Integration/Sys2 - Structural System/Sys2_Structural_System/Structural Thermal Model 2.2/STM Temperature Sensors/ Resistance Temperature Detector/Self-heat Calculation/  T vs R  ==
  data: R output; T input
function R = fcn(T)

if T >= 0
    R = 100*(1+3.9083*10^(-3)*T-5.775*10^(-7)*T^2);
else
    R = 100*(1+3.9083*10^(-3)*T-5.775*10^(-7)*T^2-4.183*10^(-12)*(T-100)*T^3);
end

== matlab function MCVT_Integration/Sys2 - Structural System/Sys2_Structural_System/Structural Thermal Model 2.2/STM Temperature Sensors/ Resistance Temperature Detector/Switch ON//OFF Control Block/Switch OFF/MATLAB Function1 ==
  data: u input; y output; Time input
function y = fcn(u,Time)

if Time>=0
    u = NaN;
end

y = u;

== matlab function MCVT_Integration/Sys2 - Structural System/Sys2_Structural_System/Structural Thermal Model 2.2/STM Temperature Sensors/ Resistance Temperature Detector/T vs R ==
  data: R output; T input
function R = fcn(T)

if T >= 0
    R = 100*(1+3.9083*10^(-3)*T-5.775*10^(-7)*T^2);
else
    R = 100*(1+3.9083*10^(-3)*T-5.775*10^(-7)*T^2-4.183*10^(-12)*(T-100)*T^3);
end

== matlab function MCVT_Integration/Sys2 - Structural System/Sys2_Structural_System/Structural Thermal Model 2.2/STM Temperature Sensors/ Resistance Temperature Detector1/Power Consumption ==
  data: Supply input; kWUsage2 output; Switch input
function kWUsage2 = Power_Consumption(Supply,Switch)

if Switch >= 1
    Voltage = 5.5; %Volts
    Current = 0.000001; %10 microAmperes
    
    kWUsage2 = (Voltage * Current)/1000;
    
    if kWUsage2 >= Supply
        kWUsage2 = Supply;
    end
    
else
    kWUsage2 = 0;
end
end

== matlab function MCVT_Integration/Sys2 - Structural System/Sys2_Structural_System/Structural Thermal Model 2.2/STM Temperature Sensors/ Resistance Temperature Detector1/R vs T ==
  data: R input; T output
function T = fcn(R)

T = 1.76520388802152e-09 * R^4 - 2.98990866289637e-07 * R^3 + 0.000987249006463873 * R^2 ...
    + 2.36178487086887 * R - 245.924688605077;

end

== matlab function MCVT_Integration/Sys 6 - Health Management and Agents/Decision Maker/Simulink Communication Modules/ECLSS_Dust//MCVT API to HMS/UDP_Send/MATLAB Function ==
  data: hi0 output; len input; hdr_Info input; rowcol input; len0 output
function [hi0, len0]=headerInfo(len, hdr_Info, rowcol)
src=hdr_Info(1);
dest=hdr_Info(2);
type=hdr_Info(3);
prio=hdr_Info(4);
row=rowcol(1); 
col=rowcol(2);
% len=0; 
hi0=[src, dest, type, prio, row, col];
len0=len;
% hi=uint8(hi);

== matlab function MCVT_Integration/Sys2 - Structural System/Sys2_Structural_System/Structural Thermal Model 2.2/STM Temperature Sensors/ Resistance Temperature Detector1/RTD with Self-heat Error/MATLAB Function ==
  data: I input; R input; U output
function U = fcn(I, R)

U = I * R;     % Operating current = 1mA
               % Voltage output U = I*R

== matlab function MCVT_Integration/Sys2 - Structural System/Sys2_Structural_System/Structural Thermal Model 2.2/STM Temperature Sensors/ Resistance Temperature Detector1/RTD without Self-heat Error/MATLAB Function ==
  data: I input; I output; R input; U output
function [I,U]= fcn(I,R)

U = I * R;     % Operating current = 1mA
               % Voltage output U = I*R

== matlab function MCVT_Integration/Sys2 - Structural System/Sys2_Structural_System/Structural Thermal Model 2.2/STM Temperature Sensors/ Resistance Temperature Detector1/Self-heat Calculation/  T vs R  ==
  data: R output; T input
function R = fcn(T)

if T >= 0
    R = 100*(1+3.9083*10^(-3)*T-5.775*10^(-7)*T^2);
else
    R = 100*(1+3.9083*10^(-3)*T-5.775*10^(-7)*T^2-4.183*10^(-12)*(T-100)*T^3);
end

== matlab function MCVT_Integration/Sys8 - Interior Environment/Habitat Interior Environment Model/Adjacent Element Data ==
  data: IEN_ADJ output
%% Manual data entry
function IEN_ADJ =Data

% HIEM_EN112
IEN_ADJ=[1	2	3	4	5	6	7	8	9	10	11	12	13	14	15	16	17	18	19	20	21	22	23	24	25	26	27	28	29	30	31	32	33	34	35	36	37	38	39	40	41	42	43	44	45	46	47	48	49	50	51	52	53	54	55	56	57	58	59	60	61	62	63	64	65	66	67	68	69	70	71	72	73	74	75	76	77	78	79	80	81	82	83	84	85	86	87	88	89	90	91	92	93	94	95	96	97	98	99	100	101	102	103	104	105	106	107	108	109	110	111	112;2 30	4	32	51	52	53	5	6	7	28	25	27	24	26	23	33	34	35	39	40	41	17	18	19	20	21	22	47	29	50	31	36	37	38	47	46	45	42	43	44	50	49	48	0	0	46	0	0	0	54	55	56	50	49	48	107	57	108	59	109	61	64	86	66	92	68	88	70	94	72	90	74	96	65	63	69	67	73	71	97	99	81	82	103	85	102	87	101	89	106	91	105	93	104	95	98	101	100	104	102	0	0	0	0	0	110	111	112	106	105	104;16	1	15	3	8	9	10	15	13	11	0	0	0	0	0	14	23	24	25	26	27	28	16	14	12	15	13	11	30	2	32	4	17	18	19	33	34	35	20	21	22	39	40	41	38	37	36	44	43	42	5	6	7	51	52	53	58	75	60	77	62	79	76	63	75	65	78	67	77	69	80	71	79	73	0	0	0	0	0	78	83	84	80	79	86	64	88	68	90	72	92	66	94	70	96	74	81	97	82	99	89	87	85	95	93	91	57	59	61	107	108	109;23	17	26	20	0	7	57	9	10	58	75	76	11	12	13	0	18	19	64	21	22	66	24	25	63	27	28	65	36	33	42	39	34	35	86	37	38	85	40	41	92	43	44	91	103	45	0	106	48	49	0	53	107	55	56	110	59	60	61	62	82	84	67	68	69	70	71	72	73	74	83	81	84	82	77	78	79	80	84	83	0	0	0	0	87	88	89	90	98	97	93	94	95	96	100	99	0	0	0	0	98	101	102	100	104	105	108	109	99	111	112	100;0	0	0	0	4	5	6	3	8	9	13	14	15	16	3	1	2	17	18	4	20	21	1	23	24	3	26	27	0	0	0	0	30	33	34	29	36	37	32	39	40	31	42	43	46	47	29	49	50	31	32	51	52	31	54	55	7	10	57	58	0	0	25	19	28	22	63	64	65	66	67	68	69	70	11	12	75	76	77	0	72	74	71	73	38	35	85	86	87	88	44	41	91	92	93	94	90	89	96	95	0	103	45	105	106	48	53	107	0	56	110	111;3	4	8	5	6	0	0	0	0	0	10	11	9	13	8	15	20	21	22	5	6	7	26	27	28	8	9	10	31	32	54	51	39	40	41	42	43	44	51	52	53	54	55	56	48	49	50	56	55	54	52	0	0	0	0	0	0	0	0	0	59	60	65	66	58	57	69	70	60	59	73	74	62	61	58	75	60	77	62	79	82	61	84	62	91	92	93	94	95	96	110	107	111	108	112	109	99	100	109	112	104	105	106	112	111	110	0	0	108	0	0	0;0	0	1	2	20	21	22	26	27	28	12	0	14	0	16	0	0	0	0	17	18	19	0	0	0	23	24	25	0	0	29	30	0	0	0	0	0	0	33	34	35	36	37	38	0	0	0	45	46	47	39	40	41	42	43	44	66	65	70	69	74	73	0	0	63	64	0	0	67	68	0	0	71	72	76	0	78	0	80	0	0	81	0	83	0	0	0	0	0	0	85	86	87	88	89	90	0	0	97	98	0	0	0	101	102	103	92	94	96	91	93	95];

end
